simulink model "V2x_TC_IntelligentTrafficSigns_cs"  (Simulink 7.6)
settings: Solver=FixedStepDiscrete  SolverMode=SingleTasking  StartTime=0.0  StopTime=66  FixedStep=1/100  RelTol=1e-3
PreLoadFcn:
  | check_NUTS_defined
  | 
  | assignin('base',['model_closed_' bdroot],false);addpath('../includes_V2x');
InitFcn:
  | PreScanExperimentInitFcn(bdroot)
StopFcn:
  | PreScanExperimentStopFcn(bdroot);
  | 
undefined symbols (not defined in the file or in its folder): acceleration, actorID, actorType, DiscIntNeverNeededParam, GPS_alt, GPS_lat, GPS_long, lightBarInUse, messageID, Newton, publicActorType, Raphson, rotZ, sigbuilder_block, sireneInUse, trafficLightPriority, turnAdvice, velocity, x_ini_VRML, y_ini_VRML, yawRate, z_ini_VRML
libraries: simulink x111, PreScanSimulinkLibrary x7, simulink_extras x4, PreScanUsefulBlocks x3
(part 3 of 16)

== system V2x_TC_IntelligentTrafficSigns_cs/TS_Tcross_2 ==
  CAM generation time step : Constant  Value=0.1
  CAM repetition time step : Constant  Value=0.05
  Cartesian to GPS : Subsystem
  Green light : Constant  Value=0
  Ground_{1..5} (5 alike) : Ground
  Infrastructure CAM generator : Subsystem
  Orange light : Constant  Value=0
  Red light : Constant  Value=0
  Tx_Tcross_2_BusAssignment : BusAssignment  Tag=BusAntennaT  AssignedSignals=warning,messageID,actorID,actorType,trafficLightPriority,GPS_lat,GPS_long,GPS_alt,rotZ
  Tx_Tcross_2_Mux : Subsystem  Tag=MuxAntennaT  mask(PreScan Mux Block:){Channel=1, TimeStep=1/100}
      mask prompts: TimeStep "Time Step [s]"
      settings: DeleteFcn=PreScanBlockDeleteFcn;
      mask Display:
      | fprintf('Channel: %d',Channel)
      | port_label('input', 1, 'Warning signal ID')
      | port_label('input', 2, 'Maneuvre ID')
      | port_label('input', 3, 'Vehicle Type')
      | port_label('input', 4, 'Braking Capacity')
      | port_label('input', 5, 'Vehicle Size')
      | port_label('input', 6, 'spare')
  X : Constant  Value=-13.5383873
  Y : Constant  Value=-6.6508584
  Z : Constant  Value=0
  actorID : Constant  Value=112
  actorType : Constant  Value=14
  messageID : Constant  Value=905
  rotZ : Constant  Value=270
  warning : Constant  Value=0
  Tx_Tcross_2 : Outport  Tag=AntennaTOutport
  wires:
  CAM generation time step/1 -> Infrastructure CAM generator/1(CAM gen time step)
  CAM repetition time step/1 -> Infrastructure CAM generator/2(CAM rep time step)
  warning/1 -> Infrastructure CAM generator/3(warning)
  messageID/1 -> Infrastructure CAM generator/4(messageID)
  actorID/1 -> Infrastructure CAM generator/5(actorID)
  actorType/1 -> Infrastructure CAM generator/6(actorType)
  Red light/1 -> Infrastructure CAM generator/7(Red light)
  Orange light/1 -> Infrastructure CAM generator/8(Orange light)
  Green light/1 -> Infrastructure CAM generator/9(Green light)
  rotZ/1 -> Infrastructure CAM generator/13(rotZ)
  X/1 -> Cartesian to GPS/1(X)
  Y/1 -> Cartesian to GPS/2(Y)
  Z/1 -> Cartesian to GPS/3(Z)
  Cartesian to GPS/1(lat) -> Infrastructure CAM generator/10(GPS_lat)
  Cartesian to GPS/2(long) -> Infrastructure CAM generator/11(GPS_long)
  Cartesian to GPS/3(alt) -> Infrastructure CAM generator/12(GPS_alt)
  Tx_Tcross_2_Mux/2(Out2) -> [Tx_Tcross_2]
  [Tx_Tcross_2] -> Tx_Tcross_2_BusAssignment/1
  Tx_Tcross_2_BusAssignment/1 -> Tx_Tcross_2_Mux/6(spare)
  Tx_Tcross_2_Mux/1(Out1) -> Tx_Tcross_2/1
  Infrastructure CAM generator/9(CAM_rotZ) -> Tx_Tcross_2_BusAssignment/10
  Infrastructure CAM generator/8(CAM_GPSalt) -> Tx_Tcross_2_BusAssignment/9
  Infrastructure CAM generator/7(CAM_GPSlong) -> Tx_Tcross_2_BusAssignment/8
  Infrastructure CAM generator/6(CAM_GPSlat) -> Tx_Tcross_2_BusAssignment/7
  Infrastructure CAM generator/5(CAM_trafficLightPriority) -> Tx_Tcross_2_BusAssignment/6
  Infrastructure CAM generator/4(CAM_vehicleType) -> Tx_Tcross_2_BusAssignment/5
  Infrastructure CAM generator/3(CAM_vehicleID) -> Tx_Tcross_2_BusAssignment/4
  Infrastructure CAM generator/2(CAM_messageID) -> Tx_Tcross_2_BusAssignment/3
  Infrastructure CAM generator/1(CAM_warning) -> Tx_Tcross_2_BusAssignment/2
  Ground_1/1 -> Tx_Tcross_2_Mux/1(warning_signal_id)
  Ground_2/1 -> Tx_Tcross_2_Mux/2(maneuvre_id)
  Ground_3/1 -> Tx_Tcross_2_Mux/3(vehicle_type)
  Ground_4/1 -> Tx_Tcross_2_Mux/4(brakingcapacity)
  Ground_5/1 -> Tx_Tcross_2_Mux/5(vehicle_size)

== system V2x_TC_IntelligentTrafficSigns_cs/TS_Tcross_3 ==
  CAM generation time step : Constant  Value=0.1
  CAM repetition time step : Constant  Value=0.05
  Cartesian to GPS : Subsystem
  Green light : Constant  Value=0
  Ground_{1..5} (5 alike) : Ground
  Infrastructure CAM generator : Subsystem
  Orange light : Constant  Value=0
  Red light : Constant  Value=0
  Tx_Tcross_3_BusAssignment : BusAssignment  Tag=BusAntennaT  AssignedSignals=warning,messageID,actorID,actorType,trafficLightPriority,GPS_lat,GPS_long,GPS_alt,rotZ
  Tx_Tcross_3_Mux : Subsystem  Tag=MuxAntennaT  mask(PreScan Mux Block:){Channel=1, TimeStep=1/100}
      mask prompts: TimeStep "Time Step [s]"
      settings: DeleteFcn=PreScanBlockDeleteFcn;
      mask Display:
      | fprintf('Channel: %d',Channel)
      | port_label('input', 1, 'Warning signal ID')
      | port_label('input', 2, 'Maneuvre ID')
      | port_label('input', 3, 'Vehicle Type')
      | port_label('input', 4, 'Braking Capacity')
      | port_label('input', 5, 'Vehicle Size')
      | port_label('input', 6, 'spare')
  X : Constant  Value=-22.7751579
  Y : Constant  Value=6.28594971
  Z : Constant  Value=0
  actorID : Constant  Value=19
  actorType : Constant  Value=14
  messageID : Constant  Value=905
  rotZ : Constant  Value=90
  warning : Constant  Value=0
  Tx_Tcross_3 : Outport  Tag=AntennaTOutport
  wires:
  CAM generation time step/1 -> Infrastructure CAM generator/1(CAM gen time step)
  CAM repetition time step/1 -> Infrastructure CAM generator/2(CAM rep time step)
  warning/1 -> Infrastructure CAM generator/3(warning)
  messageID/1 -> Infrastructure CAM generator/4(messageID)
  actorID/1 -> Infrastructure CAM generator/5(actorID)
  actorType/1 -> Infrastructure CAM generator/6(actorType)
  Red light/1 -> Infrastructure CAM generator/7(Red light)
  Orange light/1 -> Infrastructure CAM generator/8(Orange light)
  Green light/1 -> Infrastructure CAM generator/9(Green light)
  rotZ/1 -> Infrastructure CAM generator/13(rotZ)
  X/1 -> Cartesian to GPS/1(X)
  Y/1 -> Cartesian to GPS/2(Y)
  Z/1 -> Cartesian to GPS/3(Z)
  Cartesian to GPS/1(lat) -> Infrastructure CAM generator/10(GPS_lat)
  Cartesian to GPS/2(long) -> Infrastructure CAM generator/11(GPS_long)
  Cartesian to GPS/3(alt) -> Infrastructure CAM generator/12(GPS_alt)
  Tx_Tcross_3_Mux/2(Out2) -> [Tx_Tcross_3]
  [Tx_Tcross_3] -> Tx_Tcross_3_BusAssignment/1
  Tx_Tcross_3_BusAssignment/1 -> Tx_Tcross_3_Mux/6(spare)
  Tx_Tcross_3_Mux/1(Out1) -> Tx_Tcross_3/1
  Infrastructure CAM generator/9(CAM_rotZ) -> Tx_Tcross_3_BusAssignment/10
  Infrastructure CAM generator/8(CAM_GPSalt) -> Tx_Tcross_3_BusAssignment/9
  Infrastructure CAM generator/7(CAM_GPSlong) -> Tx_Tcross_3_BusAssignment/8
  Infrastructure CAM generator/6(CAM_GPSlat) -> Tx_Tcross_3_BusAssignment/7
  Infrastructure CAM generator/5(CAM_trafficLightPriority) -> Tx_Tcross_3_BusAssignment/6
  Infrastructure CAM generator/4(CAM_vehicleType) -> Tx_Tcross_3_BusAssignment/5
  Infrastructure CAM generator/3(CAM_vehicleID) -> Tx_Tcross_3_BusAssignment/4
  Infrastructure CAM generator/2(CAM_messageID) -> Tx_Tcross_3_BusAssignment/3
  Infrastructure CAM generator/1(CAM_warning) -> Tx_Tcross_3_BusAssignment/2
  Ground_1/1 -> Tx_Tcross_3_Mux/1(warning_signal_id)
  Ground_2/1 -> Tx_Tcross_3_Mux/2(maneuvre_id)
  Ground_3/1 -> Tx_Tcross_3_Mux/3(vehicle_type)
  Ground_4/1 -> Tx_Tcross_3_Mux/4(brakingcapacity)
  Ground_5/1 -> Tx_Tcross_3_Mux/5(vehicle_size)

== system V2x_TC_IntelligentTrafficSigns_cs/TS_end_restrictions ==
  CAM generation time step : Constant  Value=0.1
  CAM repetition time step : Constant  Value=0.05
  Cartesian to GPS : Subsystem
  Green light : Constant  Value=0
  Ground_{1..5} (5 alike) : Ground
  Infrastructure CAM generator : Subsystem
  Orange light : Constant  Value=0
  Red light : Constant  Value=0
  Tx_end_restrictions_BusAssignment : BusAssignment  Tag=BusAntennaT  AssignedSignals=warning,messageID,actorID,actorType,trafficLightPriority,GPS_lat,GPS_long,GPS_alt,rotZ
  Tx_end_restrictions_Mux : Subsystem  Tag=MuxAntennaT  mask(PreScan Mux Block:){Channel=1, TimeStep=1/100}
      mask prompts: TimeStep "Time Step [s]"
      settings: DeleteFcn=PreScanBlockDeleteFcn;
      mask Display:
      | fprintf('Channel: %d',Channel)
      | port_label('input', 1, 'Warning signal ID')
      | port_label('input', 2, 'Maneuvre ID')
      | port_label('input', 3, 'Vehicle Type')
      | port_label('input', 4, 'Braking Capacity')
      | port_label('input', 5, 'Vehicle Size')
      | port_label('input', 6, 'spare')
  X : Constant  Value=-182.918945
  Y : Constant  Value=-63.2720566
  Z : Constant  Value=0
  actorID : Constant  Value=25
  actorType : Constant  Value=14
  messageID : Constant  Value=901
  rotZ : Constant  Value=270
  warning : Constant  Value=0
  Tx_end_restrictions : Outport  Tag=AntennaTOutport
  wires:
  CAM generation time step/1 -> Infrastructure CAM generator/1(CAM gen time step)
  CAM repetition time step/1 -> Infrastructure CAM generator/2(CAM rep time step)
  warning/1 -> Infrastructure CAM generator/3(warning)
  messageID/1 -> Infrastructure CAM generator/4(messageID)
  actorID/1 -> Infrastructure CAM generator/5(actorID)
  actorType/1 -> Infrastructure CAM generator/6(actorType)
  Red light/1 -> Infrastructure CAM generator/7(Red light)
  Orange light/1 -> Infrastructure CAM generator/8(Orange light)
  Green light/1 -> Infrastructure CAM generator/9(Green light)
  rotZ/1 -> Infrastructure CAM generator/13(rotZ)
  X/1 -> Cartesian to GPS/1(X)
  Y/1 -> Cartesian to GPS/2(Y)
  Z/1 -> Cartesian to GPS/3(Z)
  Cartesian to GPS/1(lat) -> Infrastructure CAM generator/10(GPS_lat)
  Cartesian to GPS/2(long) -> Infrastructure CAM generator/11(GPS_long)
  Cartesian to GPS/3(alt) -> Infrastructure CAM generator/12(GPS_alt)
  Tx_end_restrictions_Mux/2(Out2) -> [Tx_end_restrictions]
  [Tx_end_restrictions] -> Tx_end_restrictions_BusAssignment/1
  Tx_end_restrictions_BusAssignment/1 -> Tx_end_restrictions_Mux/6(spare)
  Tx_end_restrictions_Mux/1(Out1) -> Tx_end_restrictions/1
  Infrastructure CAM generator/9(CAM_rotZ) -> Tx_end_restrictions_BusAssignment/10
  Infrastructure CAM generator/8(CAM_GPSalt) -> Tx_end_restrictions_BusAssignment/9
  Infrastructure CAM generator/7(CAM_GPSlong) -> Tx_end_restrictions_BusAssignment/8
  Infrastructure CAM generator/6(CAM_GPSlat) -> Tx_end_restrictions_BusAssignment/7
  Infrastructure CAM generator/5(CAM_trafficLightPriority) -> Tx_end_restrictions_BusAssignment/6
  Infrastructure CAM generator/4(CAM_vehicleType) -> Tx_end_restrictions_BusAssignment/5
  Infrastructure CAM generator/3(CAM_vehicleID) -> Tx_end_restrictions_BusAssignment/4
  Infrastructure CAM generator/2(CAM_messageID) -> Tx_end_restrictions_BusAssignment/3
  Infrastructure CAM generator/1(CAM_warning) -> Tx_end_restrictions_BusAssignment/2
  Ground_1/1 -> Tx_end_restrictions_Mux/1(warning_signal_id)
  Ground_2/1 -> Tx_end_restrictions_Mux/2(maneuvre_id)
  Ground_3/1 -> Tx_end_restrictions_Mux/3(vehicle_type)
  Ground_4/1 -> Tx_end_restrictions_Mux/4(brakingcapacity)
  Ground_5/1 -> Tx_end_restrictions_Mux/5(vehicle_size)

== system V2x_TC_IntelligentTrafficSigns_cs/TS_end_restrictions_r ==
  CAM generation time step : Constant  Value=0.1
  CAM repetition time step : Constant  Value=0.05
  Cartesian to GPS : Subsystem
  Green light : Constant  Value=0
  Ground_{1..5} (5 alike) : Ground
  Infrastructure CAM generator : Subsystem
  Orange light : Constant  Value=0
  Red light : Constant  Value=0
  Tx_end_restrictions_r_BusAssignment : BusAssignment  Tag=BusAntennaT  AssignedSignals=warning,messageID,actorID,actorType,trafficLightPriority,GPS_lat,GPS_long,GPS_alt,rotZ
  Tx_end_restrictions_r_Mux : Subsystem  Tag=MuxAntennaT  mask(PreScan Mux Block:){Channel=1, TimeStep=1/100}
      mask prompts: TimeStep "Time Step [s]"
      settings: DeleteFcn=PreScanBlockDeleteFcn;
      mask Display:
      | fprintf('Channel: %d',Channel)
      | port_label('input', 1, 'Warning signal ID')
      | port_label('input', 2, 'Maneuvre ID')
      | port_label('input', 3, 'Vehicle Type')
      | port_label('input', 4, 'Braking Capacity')
      | port_label('input', 5, 'Vehicle Size')
      | port_label('input', 6, 'spare')
  X : Constant  Value=-23.4024277
  Y : Constant  Value=48.67223
  Z : Constant  Value=0
  actorID : Constant  Value=8
  actorType : Constant  Value=14
  messageID : Constant  Value=901
  rotZ : Constant  Value=90
  warning : Constant  Value=0
  Tx_end_restrictions_r : Outport  Tag=AntennaTOutport
  wires:
  CAM generation time step/1 -> Infrastructure CAM generator/1(CAM gen time step)
  CAM repetition time step/1 -> Infrastructure CAM generator/2(CAM rep time step)
  warning/1 -> Infrastructure CAM generator/3(warning)
  messageID/1 -> Infrastructure CAM generator/4(messageID)
  actorID/1 -> Infrastructure CAM generator/5(actorID)
  actorType/1 -> Infrastructure CAM generator/6(actorType)
  Red light/1 -> Infrastructure CAM generator/7(Red light)
  Orange light/1 -> Infrastructure CAM generator/8(Orange light)
  Green light/1 -> Infrastructure CAM generator/9(Green light)
  rotZ/1 -> Infrastructure CAM generator/13(rotZ)
  X/1 -> Cartesian to GPS/1(X)
  Y/1 -> Cartesian to GPS/2(Y)
  Z/1 -> Cartesian to GPS/3(Z)
  Cartesian to GPS/1(lat) -> Infrastructure CAM generator/10(GPS_lat)
  Cartesian to GPS/2(long) -> Infrastructure CAM generator/11(GPS_long)
  Cartesian to GPS/3(alt) -> Infrastructure CAM generator/12(GPS_alt)
  Tx_end_restrictions_r_Mux/2(Out2) -> [Tx_end_restrictions_r]
  [Tx_end_restrictions_r] -> Tx_end_restrictions_r_BusAssignment/1
  Tx_end_restrictions_r_BusAssignment/1 -> Tx_end_restrictions_r_Mux/6(spare)
  Tx_end_restrictions_r_Mux/1(Out1) -> Tx_end_restrictions_r/1
  Infrastructure CAM generator/9(CAM_rotZ) -> Tx_end_restrictions_r_BusAssignment/10
  Infrastructure CAM generator/8(CAM_GPSalt) -> Tx_end_restrictions_r_BusAssignment/9
  Infrastructure CAM generator/7(CAM_GPSlong) -> Tx_end_restrictions_r_BusAssignment/8
  Infrastructure CAM generator/6(CAM_GPSlat) -> Tx_end_restrictions_r_BusAssignment/7
  Infrastructure CAM generator/5(CAM_trafficLightPriority) -> Tx_end_restrictions_r_BusAssignment/6
  Infrastructure CAM generator/4(CAM_vehicleType) -> Tx_end_restrictions_r_BusAssignment/5
  Infrastructure CAM generator/3(CAM_vehicleID) -> Tx_end_restrictions_r_BusAssignment/4
  Infrastructure CAM generator/2(CAM_messageID) -> Tx_end_restrictions_r_BusAssignment/3
  Infrastructure CAM generator/1(CAM_warning) -> Tx_end_restrictions_r_BusAssignment/2
  Ground_1/1 -> Tx_end_restrictions_r_Mux/1(warning_signal_id)
  Ground_2/1 -> Tx_end_restrictions_r_Mux/2(maneuvre_id)
  Ground_3/1 -> Tx_end_restrictions_r_Mux/3(vehicle_type)
  Ground_4/1 -> Tx_end_restrictions_r_Mux/4(brakingcapacity)
  Ground_5/1 -> Tx_end_restrictions_r_Mux/5(vehicle_size)

== system V2x_TC_IntelligentTrafficSigns_cs/TS_no_entry ==
  CAM generation time step : Constant  Value=0.1
  CAM repetition time step : Constant  Value=0.05
  Cartesian to GPS : Subsystem
  Green light : Constant  Value=0
  Ground_{1..5} (5 alike) : Ground
  Infrastructure CAM generator : Subsystem
  Orange light : Constant  Value=0
  Red light : Constant  Value=0
  Tx_no_entry_BusAssignment : BusAssignment  Tag=BusAntennaT  AssignedSignals=warning,messageID,actorID,actorType,trafficLightPriority,GPS_lat,GPS_long,GPS_alt,rotZ
  Tx_no_entry_Mux : Subsystem  Tag=MuxAntennaT  mask(PreScan Mux Block:){Channel=1, TimeStep=1/100}
      mask prompts: TimeStep "Time Step [s]"
      settings: DeleteFcn=PreScanBlockDeleteFcn;
      mask Display:
      | fprintf('Channel: %d',Channel)
      | port_label('input', 1, 'Warning signal ID')
      | port_label('input', 2, 'Maneuvre ID')
      | port_label('input', 3, 'Vehicle Type')
      | port_label('input', 4, 'Braking Capacity')
      | port_label('input', 5, 'Vehicle Size')
      | port_label('input', 6, 'spare')
  X : Constant  Value=-152.7322
  Y : Constant  Value=78.54401
  Z : Constant  Value=0
  actorID : Constant  Value=22
  actorType : Constant  Value=14
  messageID : Constant  Value=903
  rotZ : Constant  Value=230
  warning : Constant  Value=0
  Tx_no_entry : Outport  Tag=AntennaTOutport
  wires:
  CAM generation time step/1 -> Infrastructure CAM generator/1(CAM gen time step)
  CAM repetition time step/1 -> Infrastructure CAM generator/2(CAM rep time step)
  warning/1 -> Infrastructure CAM generator/3(warning)
  messageID/1 -> Infrastructure CAM generator/4(messageID)
  actorID/1 -> Infrastructure CAM generator/5(actorID)
  actorType/1 -> Infrastructure CAM generator/6(actorType)
  Red light/1 -> Infrastructure CAM generator/7(Red light)
  Orange light/1 -> Infrastructure CAM generator/8(Orange light)
  Green light/1 -> Infrastructure CAM generator/9(Green light)
  rotZ/1 -> Infrastructure CAM generator/13(rotZ)
  X/1 -> Cartesian to GPS/1(X)
  Y/1 -> Cartesian to GPS/2(Y)
  Z/1 -> Cartesian to GPS/3(Z)
  Cartesian to GPS/1(lat) -> Infrastructure CAM generator/10(GPS_lat)
  Cartesian to GPS/2(long) -> Infrastructure CAM generator/11(GPS_long)
  Cartesian to GPS/3(alt) -> Infrastructure CAM generator/12(GPS_alt)
  Tx_no_entry_Mux/2(Out2) -> [Tx_no_entry]
  [Tx_no_entry] -> Tx_no_entry_BusAssignment/1
  Tx_no_entry_BusAssignment/1 -> Tx_no_entry_Mux/6(spare)
  Tx_no_entry_Mux/1(Out1) -> Tx_no_entry/1
  Infrastructure CAM generator/9(CAM_rotZ) -> Tx_no_entry_BusAssignment/10
  Infrastructure CAM generator/8(CAM_GPSalt) -> Tx_no_entry_BusAssignment/9
  Infrastructure CAM generator/7(CAM_GPSlong) -> Tx_no_entry_BusAssignment/8
  Infrastructure CAM generator/6(CAM_GPSlat) -> Tx_no_entry_BusAssignment/7
  Infrastructure CAM generator/5(CAM_trafficLightPriority) -> Tx_no_entry_BusAssignment/6
  Infrastructure CAM generator/4(CAM_vehicleType) -> Tx_no_entry_BusAssignment/5
  Infrastructure CAM generator/3(CAM_vehicleID) -> Tx_no_entry_BusAssignment/4
  Infrastructure CAM generator/2(CAM_messageID) -> Tx_no_entry_BusAssignment/3
  Infrastructure CAM generator/1(CAM_warning) -> Tx_no_entry_BusAssignment/2
  Ground_1/1 -> Tx_no_entry_Mux/1(warning_signal_id)
  Ground_2/1 -> Tx_no_entry_Mux/2(maneuvre_id)
  Ground_3/1 -> Tx_no_entry_Mux/3(vehicle_type)
  Ground_4/1 -> Tx_no_entry_Mux/4(brakingcapacity)
  Ground_5/1 -> Tx_no_entry_Mux/5(vehicle_size)

== system V2x_TC_IntelligentTrafficSigns_cs/TS_v50_1 ==
  CAM generation time step : Constant  Value=0.1
  CAM repetition time step : Constant  Value=0.05
  Cartesian to GPS : Subsystem
  Green light : Constant  Value=0
  Ground_{1..5} (5 alike) : Ground
  Infrastructure CAM generator : Subsystem
  Orange light : Constant  Value=0
  Red light : Constant  Value=0
  Tx_v50_1_BusAssignment : BusAssignment  Tag=BusAntennaT  AssignedSignals=warning,messageID,actorID,actorType,trafficLightPriority,GPS_lat,GPS_long,GPS_alt,rotZ
  Tx_v50_1_Mux : Subsystem  Tag=MuxAntennaT  mask(PreScan Mux Block:){Channel=1, TimeStep=1/100}
      mask prompts: TimeStep "Time Step [s]"
      settings: DeleteFcn=PreScanBlockDeleteFcn;
      mask Display:
      | fprintf('Channel: %d',Channel)
      | port_label('input', 1, 'Warning signal ID')
      | port_label('input', 2, 'Maneuvre ID')
      | port_label('input', 3, 'Vehicle Type')
      | port_label('input', 4, 'Braking Capacity')
      | port_label('input', 5, 'Vehicle Size')
      | port_label('input', 6, 'spare')
  X : Constant  Value=-183.9157
  Y : Constant  Value=5.246979
  Z : Constant  Value=0
  actorID : Constant  Value=6
  actorType : Constant  Value=14
  messageID : Constant  Value=1050
  rotZ : Constant  Value=270
  warning : Constant  Value=0
  Tx_v50_1 : Outport  Tag=AntennaTOutport
  wires:
  CAM generation time step/1 -> Infrastructure CAM generator/1(CAM gen time step)
  CAM repetition time step/1 -> Infrastructure CAM generator/2(CAM rep time step)
  warning/1 -> Infrastructure CAM generator/3(warning)
  messageID/1 -> Infrastructure CAM generator/4(messageID)
  actorID/1 -> Infrastructure CAM generator/5(actorID)
  actorType/1 -> Infrastructure CAM generator/6(actorType)
  Red light/1 -> Infrastructure CAM generator/7(Red light)
  Orange light/1 -> Infrastructure CAM generator/8(Orange light)
  Green light/1 -> Infrastructure CAM generator/9(Green light)
  rotZ/1 -> Infrastructure CAM generator/13(rotZ)
  X/1 -> Cartesian to GPS/1(X)
  Y/1 -> Cartesian to GPS/2(Y)
  Z/1 -> Cartesian to GPS/3(Z)
  Cartesian to GPS/1(lat) -> Infrastructure CAM generator/10(GPS_lat)
  Cartesian to GPS/2(long) -> Infrastructure CAM generator/11(GPS_long)
  Cartesian to GPS/3(alt) -> Infrastructure CAM generator/12(GPS_alt)
  Tx_v50_1_Mux/2(Out2) -> [Tx_v50_1]
  [Tx_v50_1] -> Tx_v50_1_BusAssignment/1
  Tx_v50_1_BusAssignment/1 -> Tx_v50_1_Mux/6(spare)
  Tx_v50_1_Mux/1(Out1) -> Tx_v50_1/1
  Infrastructure CAM generator/9(CAM_rotZ) -> Tx_v50_1_BusAssignment/10
  Infrastructure CAM generator/8(CAM_GPSalt) -> Tx_v50_1_BusAssignment/9
  Infrastructure CAM generator/7(CAM_GPSlong) -> Tx_v50_1_BusAssignment/8
  Infrastructure CAM generator/6(CAM_GPSlat) -> Tx_v50_1_BusAssignment/7
  Infrastructure CAM generator/5(CAM_trafficLightPriority) -> Tx_v50_1_BusAssignment/6
  Infrastructure CAM generator/4(CAM_vehicleType) -> Tx_v50_1_BusAssignment/5
  Infrastructure CAM generator/3(CAM_vehicleID) -> Tx_v50_1_BusAssignment/4
  Infrastructure CAM generator/2(CAM_messageID) -> Tx_v50_1_BusAssignment/3
  Infrastructure CAM generator/1(CAM_warning) -> Tx_v50_1_BusAssignment/2
  Ground_1/1 -> Tx_v50_1_Mux/1(warning_signal_id)
  Ground_2/1 -> Tx_v50_1_Mux/2(maneuvre_id)
  Ground_3/1 -> Tx_v50_1_Mux/3(vehicle_type)
  Ground_4/1 -> Tx_v50_1_Mux/4(brakingcapacity)
  Ground_5/1 -> Tx_v50_1_Mux/5(vehicle_size)

== system V2x_TC_IntelligentTrafficSigns_cs/TS_v50_2 ==
  CAM generation time step : Constant  Value=0.1
  CAM repetition time step : Constant  Value=0.05
  Cartesian to GPS : Subsystem
  Green light : Constant  Value=0
  Ground_{1..5} (5 alike) : Ground
  Infrastructure CAM generator : Subsystem
  Orange light : Constant  Value=0
  Red light : Constant  Value=0
  Tx_v50_2_BusAssignment : BusAssignment  Tag=BusAntennaT  AssignedSignals=warning,messageID,actorID,actorType,trafficLightPriority,GPS_lat,GPS_long,GPS_alt,rotZ
  Tx_v50_2_Mux : Subsystem  Tag=MuxAntennaT  mask(PreScan Mux Block:){Channel=1, TimeStep=1/100}
      mask prompts: TimeStep "Time Step [s]"
      settings: DeleteFcn=PreScanBlockDeleteFcn;
      mask Display:
      | fprintf('Channel: %d',Channel)
      | port_label('input', 1, 'Warning signal ID')
      | port_label('input', 2, 'Maneuvre ID')
      | port_label('input', 3, 'Vehicle Type')
      | port_label('input', 4, 'Braking Capacity')
      | port_label('input', 5, 'Vehicle Size')
      | port_label('input', 6, 'spare')
  X : Constant  Value=-158.218979
  Y : Constant  Value=87.64631
  Z : Constant  Value=0
  actorID : Constant  Value=20
  actorType : Constant  Value=14
  messageID : Constant  Value=1050
  rotZ : Constant  Value=20
  warning : Constant  Value=0
  Tx_v50_2 : Outport  Tag=AntennaTOutport
  wires:
  CAM generation time step/1 -> Infrastructure CAM generator/1(CAM gen time step)
  CAM repetition time step/1 -> Infrastructure CAM generator/2(CAM rep time step)
  warning/1 -> Infrastructure CAM generator/3(warning)
  messageID/1 -> Infrastructure CAM generator/4(messageID)
  actorID/1 -> Infrastructure CAM generator/5(actorID)
  actorType/1 -> Infrastructure CAM generator/6(actorType)
  Red light/1 -> Infrastructure CAM generator/7(Red light)
  Orange light/1 -> Infrastructure CAM generator/8(Orange light)
  Green light/1 -> Infrastructure CAM generator/9(Green light)
  rotZ/1 -> Infrastructure CAM generator/13(rotZ)
  X/1 -> Cartesian to GPS/1(X)
  Y/1 -> Cartesian to GPS/2(Y)
  Z/1 -> Cartesian to GPS/3(Z)
  Cartesian to GPS/1(lat) -> Infrastructure CAM generator/10(GPS_lat)
  Cartesian to GPS/2(long) -> Infrastructure CAM generator/11(GPS_long)
  Cartesian to GPS/3(alt) -> Infrastructure CAM generator/12(GPS_alt)
  Tx_v50_2_Mux/2(Out2) -> [Tx_v50_2]
  [Tx_v50_2] -> Tx_v50_2_BusAssignment/1
  Tx_v50_2_BusAssignment/1 -> Tx_v50_2_Mux/6(spare)
  Tx_v50_2_Mux/1(Out1) -> Tx_v50_2/1
  Infrastructure CAM generator/9(CAM_rotZ) -> Tx_v50_2_BusAssignment/10
  Infrastructure CAM generator/8(CAM_GPSalt) -> Tx_v50_2_BusAssignment/9
  Infrastructure CAM generator/7(CAM_GPSlong) -> Tx_v50_2_BusAssignment/8
  Infrastructure CAM generator/6(CAM_GPSlat) -> Tx_v50_2_BusAssignment/7
  Infrastructure CAM generator/5(CAM_trafficLightPriority) -> Tx_v50_2_BusAssignment/6
  Infrastructure CAM generator/4(CAM_vehicleType) -> Tx_v50_2_BusAssignment/5
  Infrastructure CAM generator/3(CAM_vehicleID) -> Tx_v50_2_BusAssignment/4
  Infrastructure CAM generator/2(CAM_messageID) -> Tx_v50_2_BusAssignment/3
  Infrastructure CAM generator/1(CAM_warning) -> Tx_v50_2_BusAssignment/2
  Ground_1/1 -> Tx_v50_2_Mux/1(warning_signal_id)
  Ground_2/1 -> Tx_v50_2_Mux/2(maneuvre_id)
  Ground_3/1 -> Tx_v50_2_Mux/3(vehicle_type)
  Ground_4/1 -> Tx_v50_2_Mux/4(brakingcapacity)
  Ground_5/1 -> Tx_v50_2_Mux/5(vehicle_size)

== system V2x_TC_IntelligentTrafficSigns_cs/TS_v50_3 ==
  CAM generation time step : Constant  Value=0.1
  CAM repetition time step : Constant  Value=0.05
  Cartesian to GPS : Subsystem
  Green light : Constant  Value=0
  Ground_{1..5} (5 alike) : Ground
  Infrastructure CAM generator : Subsystem
  Orange light : Constant  Value=0
  Red light : Constant  Value=0
  Tx_v50_3_BusAssignment : BusAssignment  Tag=BusAntennaT  AssignedSignals=warning,messageID,actorID,actorType,trafficLightPriority,GPS_lat,GPS_long,GPS_alt,rotZ
  Tx_v50_3_Mux : Subsystem  Tag=MuxAntennaT  mask(PreScan Mux Block:){Channel=1, TimeStep=1/100}
      mask prompts: TimeStep "Time Step [s]"
      settings: DeleteFcn=PreScanBlockDeleteFcn;
      mask Display:
      | fprintf('Channel: %d',Channel)
      | port_label('input', 1, 'Warning signal ID')
      | port_label('input', 2, 'Maneuvre ID')
      | port_label('input', 3, 'Vehicle Type')
      | port_label('input', 4, 'Braking Capacity')
      | port_label('input', 5, 'Vehicle Size')
      | port_label('input', 6, 'spare')
  X : Constant  Value=-232.3668
  Y : Constant  Value=84.76238
  Z : Constant  Value=0
  actorID : Constant  Value=33
  actorType : Constant  Value=14
  messageID : Constant  Value=1050
  rotZ : Constant  Value=140
  warning : Constant  Value=0
  Tx_v50_3 : Outport  Tag=AntennaTOutport
  wires:
  CAM generation time step/1 -> Infrastructure CAM generator/1(CAM gen time step)
  CAM repetition time step/1 -> Infrastructure CAM generator/2(CAM rep time step)
  warning/1 -> Infrastructure CAM generator/3(warning)
  messageID/1 -> Infrastructure CAM generator/4(messageID)
  actorID/1 -> Infrastructure CAM generator/5(actorID)
  actorType/1 -> Infrastructure CAM generator/6(actorType)
  Red light/1 -> Infrastructure CAM generator/7(Red light)
  Orange light/1 -> Infrastructure CAM generator/8(Orange light)
  Green light/1 -> Infrastructure CAM generator/9(Green light)
  rotZ/1 -> Infrastructure CAM generator/13(rotZ)
  X/1 -> Cartesian to GPS/1(X)
  Y/1 -> Cartesian to GPS/2(Y)
  Z/1 -> Cartesian to GPS/3(Z)
  Cartesian to GPS/1(lat) -> Infrastructure CAM generator/10(GPS_lat)
  Cartesian to GPS/2(long) -> Infrastructure CAM generator/11(GPS_long)
  Cartesian to GPS/3(alt) -> Infrastructure CAM generator/12(GPS_alt)
  Tx_v50_3_Mux/2(Out2) -> [Tx_v50_3]
  [Tx_v50_3] -> Tx_v50_3_BusAssignment/1
  Tx_v50_3_BusAssignment/1 -> Tx_v50_3_Mux/6(spare)
  Tx_v50_3_Mux/1(Out1) -> Tx_v50_3/1
  Infrastructure CAM generator/9(CAM_rotZ) -> Tx_v50_3_BusAssignment/10
  Infrastructure CAM generator/8(CAM_GPSalt) -> Tx_v50_3_BusAssignment/9
  Infrastructure CAM generator/7(CAM_GPSlong) -> Tx_v50_3_BusAssignment/8
  Infrastructure CAM generator/6(CAM_GPSlat) -> Tx_v50_3_BusAssignment/7
  Infrastructure CAM generator/5(CAM_trafficLightPriority) -> Tx_v50_3_BusAssignment/6
  Infrastructure CAM generator/4(CAM_vehicleType) -> Tx_v50_3_BusAssignment/5
  Infrastructure CAM generator/3(CAM_vehicleID) -> Tx_v50_3_BusAssignment/4
  Infrastructure CAM generator/2(CAM_messageID) -> Tx_v50_3_BusAssignment/3
  Infrastructure CAM generator/1(CAM_warning) -> Tx_v50_3_BusAssignment/2
  Ground_1/1 -> Tx_v50_3_Mux/1(warning_signal_id)
  Ground_2/1 -> Tx_v50_3_Mux/2(maneuvre_id)
  Ground_3/1 -> Tx_v50_3_Mux/3(vehicle_type)
  Ground_4/1 -> Tx_v50_3_Mux/4(brakingcapacity)
  Ground_5/1 -> Tx_v50_3_Mux/5(vehicle_size)

== system V2x_TC_IntelligentTrafficSigns_cs/TS_v80_1 ==
  CAM generation time step : Constant  Value=0.1
  CAM repetition time step : Constant  Value=0.05
  Cartesian to GPS : Subsystem
  Green light : Constant  Value=0
  Ground_{1..5} (5 alike) : Ground
  Infrastructure CAM generator : Subsystem
  Orange light : Constant  Value=0
  Red light : Constant  Value=0
  Tx_v80_1_BusAssignment : BusAssignment  Tag=BusAntennaT  AssignedSignals=warning,messageID,actorID,actorType,trafficLightPriority,GPS_lat,GPS_long,GPS_alt,rotZ
  Tx_v80_1_Mux : Subsystem  Tag=MuxAntennaT  mask(PreScan Mux Block:){Channel=1, TimeStep=1/100}
      mask prompts: TimeStep "Time Step [s]"
      settings: DeleteFcn=PreScanBlockDeleteFcn;
      mask Display:
      | fprintf('Channel: %d',Channel)
      | port_label('input', 1, 'Warning signal ID')
      | port_label('input', 2, 'Maneuvre ID')
      | port_label('input', 3, 'Vehicle Type')
      | port_label('input', 4, 'Braking Capacity')
      | port_label('input', 5, 'Vehicle Size')
      | port_label('input', 6, 'spare')
  X : Constant  Value=-194.239822
  Y : Constant  Value=4.94479942
  Z : Constant  Value=0
  actorID : Constant  Value=7
  actorType : Constant  Value=14
  messageID : Constant  Value=1080
  rotZ : Constant  Value=90
  warning : Constant  Value=0
  Tx_v80_1 : Outport  Tag=AntennaTOutport
  wires:
  CAM generation time step/1 -> Infrastructure CAM generator/1(CAM gen time step)
  CAM repetition time step/1 -> Infrastructure CAM generator/2(CAM rep time step)
  warning/1 -> Infrastructure CAM generator/3(warning)
  messageID/1 -> Infrastructure CAM generator/4(messageID)
  actorID/1 -> Infrastructure CAM generator/5(actorID)
  actorType/1 -> Infrastructure CAM generator/6(actorType)
  Red light/1 -> Infrastructure CAM generator/7(Red light)
  Orange light/1 -> Infrastructure CAM generator/8(Orange light)
  Green light/1 -> Infrastructure CAM generator/9(Green light)
  rotZ/1 -> Infrastructure CAM generator/13(rotZ)
  Cartesian to GPS/1(lat) -> Infrastructure CAM generator/10(GPS_lat)
  Cartesian to GPS/2(long) -> Infrastructure CAM generator/11(GPS_long)
  Cartesian to GPS/3(alt) -> Infrastructure CAM generator/12(GPS_alt)
  X/1 -> Cartesian to GPS/1(X)
  Y/1 -> Cartesian to GPS/2(Y)
  Z/1 -> Cartesian to GPS/3(Z)
  Tx_v80_1_Mux/2(Out2) -> [Tx_v80_1]
  [Tx_v80_1] -> Tx_v80_1_BusAssignment/1
  Tx_v80_1_BusAssignment/1 -> Tx_v80_1_Mux/6(spare)
  Tx_v80_1_Mux/1(Out1) -> Tx_v80_1/1
  Infrastructure CAM generator/9(CAM_rotZ) -> Tx_v80_1_BusAssignment/10
  Infrastructure CAM generator/8(CAM_GPSalt) -> Tx_v80_1_BusAssignment/9
  Infrastructure CAM generator/7(CAM_GPSlong) -> Tx_v80_1_BusAssignment/8
  Infrastructure CAM generator/6(CAM_GPSlat) -> Tx_v80_1_BusAssignment/7
  Infrastructure CAM generator/5(CAM_trafficLightPriority) -> Tx_v80_1_BusAssignment/6
  Infrastructure CAM generator/4(CAM_vehicleType) -> Tx_v80_1_BusAssignment/5
  Infrastructure CAM generator/3(CAM_vehicleID) -> Tx_v80_1_BusAssignment/4
  Infrastructure CAM generator/2(CAM_messageID) -> Tx_v80_1_BusAssignment/3
  Infrastructure CAM generator/1(CAM_warning) -> Tx_v80_1_BusAssignment/2
  Ground_1/1 -> Tx_v80_1_Mux/1(warning_signal_id)
  Ground_2/1 -> Tx_v80_1_Mux/2(maneuvre_id)
  Ground_3/1 -> Tx_v80_1_Mux/3(vehicle_type)
  Ground_4/1 -> Tx_v80_1_Mux/4(brakingcapacity)
  Ground_5/1 -> Tx_v80_1_Mux/5(vehicle_size)

== system V2x_TC_IntelligentTrafficSigns_cs/TS_v80_3 ==
  CAM generation time step : Constant  Value=0.1
  CAM repetition time step : Constant  Value=0.05
  Cartesian to GPS : Subsystem
  Green light : Constant  Value=0
  Ground_{1..5} (5 alike) : Ground
  Infrastructure CAM generator : Subsystem
  Orange light : Constant  Value=0
  Red light : Constant  Value=0
  Tx_v80_3_BusAssignment : BusAssignment  Tag=BusAntennaT  AssignedSignals=warning,messageID,actorID,actorType,trafficLightPriority,GPS_lat,GPS_long,GPS_alt,rotZ
  Tx_v80_3_Mux : Subsystem  Tag=MuxAntennaT  mask(PreScan Mux Block:){Channel=1, TimeStep=1/100}
      mask prompts: TimeStep "Time Step [s]"
      settings: DeleteFcn=PreScanBlockDeleteFcn;
      mask Display:
      | fprintf('Channel: %d',Channel)
      | port_label('input', 1, 'Warning signal ID')
      | port_label('input', 2, 'Maneuvre ID')
      | port_label('input', 3, 'Vehicle Type')
      | port_label('input', 4, 'Braking Capacity')
      | port_label('input', 5, 'Vehicle Size')
      | port_label('input', 6, 'spare')
  X : Constant  Value=-227.5898
  Y : Constant  Value=92.7091446
  Z : Constant  Value=0
  actorID : Constant  Value=34
  actorType : Constant  Value=14
  messageID : Constant  Value=1080
  rotZ : Constant  Value=320
  warning : Constant  Value=0
  Tx_v80_3 : Outport  Tag=AntennaTOutport
  wires:
  CAM generation time step/1 -> Infrastructure CAM generator/1(CAM gen time step)
  CAM repetition time step/1 -> Infrastructure CAM generator/2(CAM rep time step)
  warning/1 -> Infrastructure CAM generator/3(warning)
  messageID/1 -> Infrastructure CAM generator/4(messageID)
  actorID/1 -> Infrastructure CAM generator/5(actorID)
  actorType/1 -> Infrastructure CAM generator/6(actorType)
  Red light/1 -> Infrastructure CAM generator/7(Red light)
  Orange light/1 -> Infrastructure CAM generator/8(Orange light)
  Green light/1 -> Infrastructure CAM generator/9(Green light)
  rotZ/1 -> Infrastructure CAM generator/13(rotZ)
  X/1 -> Cartesian to GPS/1(X)
  Y/1 -> Cartesian to GPS/2(Y)
  Z/1 -> Cartesian to GPS/3(Z)
  Cartesian to GPS/1(lat) -> Infrastructure CAM generator/10(GPS_lat)
  Cartesian to GPS/2(long) -> Infrastructure CAM generator/11(GPS_long)
  Cartesian to GPS/3(alt) -> Infrastructure CAM generator/12(GPS_alt)
  Tx_v80_3_Mux/2(Out2) -> [Tx_v80_3]
  [Tx_v80_3] -> Tx_v80_3_BusAssignment/1
  Tx_v80_3_BusAssignment/1 -> Tx_v80_3_Mux/6(spare)
  Tx_v80_3_Mux/1(Out1) -> Tx_v80_3/1
  Infrastructure CAM generator/9(CAM_rotZ) -> Tx_v80_3_BusAssignment/10
  Infrastructure CAM generator/8(CAM_GPSalt) -> Tx_v80_3_BusAssignment/9
  Infrastructure CAM generator/7(CAM_GPSlong) -> Tx_v80_3_BusAssignment/8
  Infrastructure CAM generator/6(CAM_GPSlat) -> Tx_v80_3_BusAssignment/7
  Infrastructure CAM generator/5(CAM_trafficLightPriority) -> Tx_v80_3_BusAssignment/6
  Infrastructure CAM generator/4(CAM_vehicleType) -> Tx_v80_3_BusAssignment/5
  Infrastructure CAM generator/3(CAM_vehicleID) -> Tx_v80_3_BusAssignment/4
  Infrastructure CAM generator/2(CAM_messageID) -> Tx_v80_3_BusAssignment/3
  Infrastructure CAM generator/1(CAM_warning) -> Tx_v80_3_BusAssignment/2
  Ground_1/1 -> Tx_v80_3_Mux/1(warning_signal_id)
  Ground_2/1 -> Tx_v80_3_Mux/2(maneuvre_id)
  Ground_3/1 -> Tx_v80_3_Mux/3(vehicle_type)
  Ground_4/1 -> Tx_v80_3_Mux/4(brakingcapacity)
  Ground_5/1 -> Tx_v80_3_Mux/5(vehicle_size)

== system V2x_TC_IntelligentTrafficSigns_cs/TS_v80_4 ==
  CAM generation time step : Constant  Value=0.1
  CAM repetition time step : Constant  Value=0.05
  Cartesian to GPS : Subsystem
  Green light : Constant  Value=0
  Ground_{1..4,15} (5 alike) : Ground
  Infrastructure CAM generator : Subsystem
  Orange light : Constant  Value=0
  Red light : Constant  Value=0
  Tx_v80_4_BusAssignment : BusAssignment  Tag=BusAntennaT  AssignedSignals=warning,messageID,actorID,actorType,trafficLightPriority,GPS_lat,GPS_long,GPS_alt,rotZ
  Tx_v80_4_Mux : Subsystem  Tag=MuxAntennaT  mask(PreScan Mux Block:){Channel=1, TimeStep=1/100}
      mask prompts: TimeStep "Time Step [s]"
      settings: DeleteFcn=PreScanBlockDeleteFcn;
      mask Display:
      | fprintf('Channel: %d',Channel)
      | port_label('input', 1, 'Warning signal ID')
      | port_label('input', 2, 'Maneuvre ID')
      | port_label('input', 3, 'Vehicle Type')
      | port_label('input', 4, 'Braking Capacity')
      | port_label('input', 5, 'Vehicle Size')
      | port_label('input', 6, 'spare')
  X : Constant  Value=-13
  Y : Constant  Value=15.1984406
  Z : Constant  Value=0
  actorID : Constant  Value=38
  actorType : Constant  Value=14
  messageID : Constant  Value=1080
  rotZ : Constant  Value=270
  warning : Constant  Value=0
  Tx_v80_4 : Outport  Tag=AntennaTOutport
  wires:
  CAM generation time step/1 -> Infrastructure CAM generator/1(CAM gen time step)
  CAM repetition time step/1 -> Infrastructure CAM generator/2(CAM rep time step)
  warning/1 -> Infrastructure CAM generator/3(warning)
  messageID/1 -> Infrastructure CAM generator/4(messageID)
  actorID/1 -> Infrastructure CAM generator/5(actorID)
  actorType/1 -> Infrastructure CAM generator/6(actorType)
  Red light/1 -> Infrastructure CAM generator/7(Red light)
  Orange light/1 -> Infrastructure CAM generator/8(Orange light)
  Green light/1 -> Infrastructure CAM generator/9(Green light)
  rotZ/1 -> Infrastructure CAM generator/13(rotZ)
  X/1 -> Cartesian to GPS/1(X)
  Y/1 -> Cartesian to GPS/2(Y)
  Z/1 -> Cartesian to GPS/3(Z)
  Cartesian to GPS/1(lat) -> Infrastructure CAM generator/10(GPS_lat)
  Cartesian to GPS/2(long) -> Infrastructure CAM generator/11(GPS_long)
  Cartesian to GPS/3(alt) -> Infrastructure CAM generator/12(GPS_alt)
  Tx_v80_4_Mux/2(Out2) -> [Tx_v80_4]
  [Tx_v80_4] -> Tx_v80_4_BusAssignment/1
  Tx_v80_4_BusAssignment/1 -> Tx_v80_4_Mux/6(spare)
  Tx_v80_4_Mux/1(Out1) -> Tx_v80_4/1
  Infrastructure CAM generator/9(CAM_rotZ) -> Tx_v80_4_BusAssignment/10
  Infrastructure CAM generator/8(CAM_GPSalt) -> Tx_v80_4_BusAssignment/9
  Infrastructure CAM generator/7(CAM_GPSlong) -> Tx_v80_4_BusAssignment/8
  Infrastructure CAM generator/6(CAM_GPSlat) -> Tx_v80_4_BusAssignment/7
  Infrastructure CAM generator/5(CAM_trafficLightPriority) -> Tx_v80_4_BusAssignment/6
  Infrastructure CAM generator/4(CAM_vehicleType) -> Tx_v80_4_BusAssignment/5
  Infrastructure CAM generator/3(CAM_vehicleID) -> Tx_v80_4_BusAssignment/4
  Infrastructure CAM generator/2(CAM_messageID) -> Tx_v80_4_BusAssignment/3
  Infrastructure CAM generator/1(CAM_warning) -> Tx_v80_4_BusAssignment/2
  Ground_15/1 -> Tx_v80_4_Mux/5(vehicle_size)
  Ground_1/1 -> Tx_v80_4_Mux/1(warning_signal_id)
  Ground_2/1 -> Tx_v80_4_Mux/2(maneuvre_id)
  Ground_3/1 -> Tx_v80_4_Mux/3(vehicle_type)
  Ground_4/1 -> Tx_v80_4_Mux/4(brakingcapacity)

== system V2x_TC_IntelligentTrafficSigns_cs/Tx_Lcurve_R14 ==
  Y : Inport
  Assignment : Assignment  IndexMode=Zero-based  SampleTime=TimeStep  IndexOptions=Starting index (dialog)  Indices=PortIndex  OutputSizes=[1 1]
  Data Store Read : DataStoreRead  DataStoreName=SimCoreActuators  SampleTime=TimeStep
  Data Store Write : DataStoreWrite  DataStoreName=SimCoreActuators
  wires:
  Assignment/1 -> Data Store Write/1
  Data Store Read/1 -> Assignment/1
  Y/1 -> Assignment/2

== system V2x_TC_IntelligentTrafficSigns_cs/Tx_Lcurve_R25 ==
  Y : Inport
  Assignment : Assignment  IndexMode=Zero-based  SampleTime=TimeStep  IndexOptions=Starting index (dialog)  Indices=PortIndex  OutputSizes=[1 1]
  Data Store Read : DataStoreRead  DataStoreName=SimCoreActuators  SampleTime=TimeStep
  Data Store Write : DataStoreWrite  DataStoreName=SimCoreActuators
  wires:
  Assignment/1 -> Data Store Write/1
  Data Store Read/1 -> Assignment/1
  Y/1 -> Assignment/2

== system V2x_TC_IntelligentTrafficSigns_cs/Tx_Lcurve_R39 ==
  Y : Inport
  Assignment : Assignment  IndexMode=Zero-based  SampleTime=TimeStep  IndexOptions=Starting index (dialog)  Indices=PortIndex  OutputSizes=[1 1]
  Data Store Read : DataStoreRead  DataStoreName=SimCoreActuators  SampleTime=TimeStep
  Data Store Write : DataStoreWrite  DataStoreName=SimCoreActuators
  wires:
  Assignment/1 -> Data Store Write/1
  Data Store Read/1 -> Assignment/1
  Y/1 -> Assignment/2

== system V2x_TC_IntelligentTrafficSigns_cs/Tx_Lcurve_R55 ==
  Y : Inport
  Assignment : Assignment  IndexMode=Zero-based  SampleTime=TimeStep  IndexOptions=Starting index (dialog)  Indices=PortIndex  OutputSizes=[1 1]
  Data Store Read : DataStoreRead  DataStoreName=SimCoreActuators  SampleTime=TimeStep
  Data Store Write : DataStoreWrite  DataStoreName=SimCoreActuators
  wires:
  Assignment/1 -> Data Store Write/1
  Data Store Read/1 -> Assignment/1
  Y/1 -> Assignment/2

== system V2x_TC_IntelligentTrafficSigns_cs/Tx_Rcurve_R14 ==
  Y : Inport
  Assignment : Assignment  IndexMode=Zero-based  SampleTime=TimeStep  IndexOptions=Starting index (dialog)  Indices=PortIndex  OutputSizes=[1 1]
  Data Store Read : DataStoreRead  DataStoreName=SimCoreActuators  SampleTime=TimeStep
  Data Store Write : DataStoreWrite  DataStoreName=SimCoreActuators
  wires:
  Assignment/1 -> Data Store Write/1
  Data Store Read/1 -> Assignment/1
  Y/1 -> Assignment/2

== system V2x_TC_IntelligentTrafficSigns_cs/Tx_Rcurve_R25 ==
  Y : Inport
  Assignment : Assignment  IndexMode=Zero-based  SampleTime=TimeStep  IndexOptions=Starting index (dialog)  Indices=PortIndex  OutputSizes=[1 1]
  Data Store Read : DataStoreRead  DataStoreName=SimCoreActuators  SampleTime=TimeStep
  Data Store Write : DataStoreWrite  DataStoreName=SimCoreActuators
  wires:
  Assignment/1 -> Data Store Write/1
  Data Store Read/1 -> Assignment/1
  Y/1 -> Assignment/2

== system V2x_TC_IntelligentTrafficSigns_cs/Tx_Rcurve_R39 ==
  Y : Inport
  Assignment : Assignment  IndexMode=Zero-based  SampleTime=TimeStep  IndexOptions=Starting index (dialog)  Indices=PortIndex  OutputSizes=[1 1]
  Data Store Read : DataStoreRead  DataStoreName=SimCoreActuators  SampleTime=TimeStep
  Data Store Write : DataStoreWrite  DataStoreName=SimCoreActuators
  wires:
  Assignment/1 -> Data Store Write/1
  Data Store Read/1 -> Assignment/1
  Y/1 -> Assignment/2

== system V2x_TC_IntelligentTrafficSigns_cs/Tx_Rcurve_R55 ==
  Y : Inport
  Assignment : Assignment  IndexMode=Zero-based  SampleTime=TimeStep  IndexOptions=Starting index (dialog)  Indices=PortIndex  OutputSizes=[1 1]
  Data Store Read : DataStoreRead  DataStoreName=SimCoreActuators  SampleTime=TimeStep
  Data Store Write : DataStoreWrite  DataStoreName=SimCoreActuators
  wires:
  Assignment/1 -> Data Store Write/1
  Data Store Read/1 -> Assignment/1
  Y/1 -> Assignment/2

== system V2x_TC_IntelligentTrafficSigns_cs/Tx_Tcross_1 ==
  Y : Inport
  Assignment : Assignment  IndexMode=Zero-based  SampleTime=TimeStep  IndexOptions=Starting index (dialog)  Indices=PortIndex  OutputSizes=[1 1]
  Data Store Read : DataStoreRead  DataStoreName=SimCoreActuators  SampleTime=TimeStep
  Data Store Write : DataStoreWrite  DataStoreName=SimCoreActuators
  wires:
  Assignment/1 -> Data Store Write/1
  Data Store Read/1 -> Assignment/1
  Y/1 -> Assignment/2

== system V2x_TC_IntelligentTrafficSigns_cs/Tx_Tcross_2 ==
  Y : Inport
  Assignment : Assignment  IndexMode=Zero-based  SampleTime=TimeStep  IndexOptions=Starting index (dialog)  Indices=PortIndex  OutputSizes=[1 1]
  Data Store Read : DataStoreRead  DataStoreName=SimCoreActuators  SampleTime=TimeStep
  Data Store Write : DataStoreWrite  DataStoreName=SimCoreActuators
  wires:
  Assignment/1 -> Data Store Write/1
  Data Store Read/1 -> Assignment/1
  Y/1 -> Assignment/2

== system V2x_TC_IntelligentTrafficSigns_cs/Tx_Tcross_3 ==
  Y : Inport
  Assignment : Assignment  IndexMode=Zero-based  SampleTime=TimeStep  IndexOptions=Starting index (dialog)  Indices=PortIndex  OutputSizes=[1 1]
  Data Store Read : DataStoreRead  DataStoreName=SimCoreActuators  SampleTime=TimeStep
  Data Store Write : DataStoreWrite  DataStoreName=SimCoreActuators
  wires:
  Assignment/1 -> Data Store Write/1
  Data Store Read/1 -> Assignment/1
  Y/1 -> Assignment/2

== system V2x_TC_IntelligentTrafficSigns_cs/Tx_end_restrictions ==
  Y : Inport
  Assignment : Assignment  IndexMode=Zero-based  SampleTime=TimeStep  IndexOptions=Starting index (dialog)  Indices=PortIndex  OutputSizes=[1 1]
  Data Store Read : DataStoreRead  DataStoreName=SimCoreActuators  SampleTime=TimeStep
  Data Store Write : DataStoreWrite  DataStoreName=SimCoreActuators
  wires:
  Assignment/1 -> Data Store Write/1
  Data Store Read/1 -> Assignment/1
  Y/1 -> Assignment/2

== system V2x_TC_IntelligentTrafficSigns_cs/Tx_end_restrictions_r ==
  Y : Inport
  Assignment : Assignment  IndexMode=Zero-based  SampleTime=TimeStep  IndexOptions=Starting index (dialog)  Indices=PortIndex  OutputSizes=[1 1]
  Data Store Read : DataStoreRead  DataStoreName=SimCoreActuators  SampleTime=TimeStep
  Data Store Write : DataStoreWrite  DataStoreName=SimCoreActuators
  wires:
  Assignment/1 -> Data Store Write/1
  Data Store Read/1 -> Assignment/1
  Y/1 -> Assignment/2

== system V2x_TC_IntelligentTrafficSigns_cs/Tx_no_entry ==
  Y : Inport
  Assignment : Assignment  IndexMode=Zero-based  SampleTime=TimeStep  IndexOptions=Starting index (dialog)  Indices=PortIndex  OutputSizes=[1 1]
  Data Store Read : DataStoreRead  DataStoreName=SimCoreActuators  SampleTime=TimeStep
  Data Store Write : DataStoreWrite  DataStoreName=SimCoreActuators
  wires:
  Assignment/1 -> Data Store Write/1
  Data Store Read/1 -> Assignment/1
  Y/1 -> Assignment/2

== system V2x_TC_IntelligentTrafficSigns_cs/Tx_v50_1 ==
  Y : Inport
  Assignment : Assignment  IndexMode=Zero-based  SampleTime=TimeStep  IndexOptions=Starting index (dialog)  Indices=PortIndex  OutputSizes=[1 1]
  Data Store Read : DataStoreRead  DataStoreName=SimCoreActuators  SampleTime=TimeStep
  Data Store Write : DataStoreWrite  DataStoreName=SimCoreActuators
  wires:
  Assignment/1 -> Data Store Write/1
  Data Store Read/1 -> Assignment/1
  Y/1 -> Assignment/2

== system V2x_TC_IntelligentTrafficSigns_cs/Tx_v50_2 ==
  Y : Inport
  Assignment : Assignment  IndexMode=Zero-based  SampleTime=TimeStep  IndexOptions=Starting index (dialog)  Indices=PortIndex  OutputSizes=[1 1]
  Data Store Read : DataStoreRead  DataStoreName=SimCoreActuators  SampleTime=TimeStep
  Data Store Write : DataStoreWrite  DataStoreName=SimCoreActuators
  wires:
  Assignment/1 -> Data Store Write/1
  Data Store Read/1 -> Assignment/1
  Y/1 -> Assignment/2

== system V2x_TC_IntelligentTrafficSigns_cs/Tx_v50_3 ==
  Y : Inport
  Assignment : Assignment  IndexMode=Zero-based  SampleTime=TimeStep  IndexOptions=Starting index (dialog)  Indices=PortIndex  OutputSizes=[1 1]
  Data Store Read : DataStoreRead  DataStoreName=SimCoreActuators  SampleTime=TimeStep
  Data Store Write : DataStoreWrite  DataStoreName=SimCoreActuators
  wires:
  Assignment/1 -> Data Store Write/1
  Data Store Read/1 -> Assignment/1
  Y/1 -> Assignment/2

== system V2x_TC_IntelligentTrafficSigns_cs/Tx_v80_1 ==
  Y : Inport
  Assignment : Assignment  IndexMode=Zero-based  SampleTime=TimeStep  IndexOptions=Starting index (dialog)  Indices=PortIndex  OutputSizes=[1 1]
  Data Store Read : DataStoreRead  DataStoreName=SimCoreActuators  SampleTime=TimeStep
  Data Store Write : DataStoreWrite  DataStoreName=SimCoreActuators
  wires:
  Assignment/1 -> Data Store Write/1
  Data Store Read/1 -> Assignment/1
  Y/1 -> Assignment/2

== system V2x_TC_IntelligentTrafficSigns_cs/Tx_v80_3 ==
  Y : Inport
  Assignment : Assignment  IndexMode=Zero-based  SampleTime=TimeStep  IndexOptions=Starting index (dialog)  Indices=PortIndex  OutputSizes=[1 1]
  Data Store Read : DataStoreRead  DataStoreName=SimCoreActuators  SampleTime=TimeStep
  Data Store Write : DataStoreWrite  DataStoreName=SimCoreActuators
  wires:
  Assignment/1 -> Data Store Write/1
  Data Store Read/1 -> Assignment/1
  Y/1 -> Assignment/2

== system V2x_TC_IntelligentTrafficSigns_cs/Tx_v80_4 ==
  Y : Inport
  Assignment : Assignment  IndexMode=Zero-based  SampleTime=TimeStep  IndexOptions=Starting index (dialog)  Indices=PortIndex  OutputSizes=[1 1]
  Data Store Read : DataStoreRead  DataStoreName=SimCoreActuators  SampleTime=TimeStep
  Data Store Write : DataStoreWrite  DataStoreName=SimCoreActuators
  wires:
  Assignment/1 -> Data Store Write/1
  Data Store Read/1 -> Assignment/1
  Y/1 -> Assignment/2
